simulink model "x64_32s_001_4ks"  (Simulink 7.4)
settings: Solver=ode45  StartTime=0.0  StopTime=100000  RelTol=1e-3
undefined symbols (not defined in the file): gcbh, ROACH, x64_32s_001_4ks_beam_mux_order_in_user_data_out, x64_32s_001_4ks_beam_mux_order_out_user_data_out, x64_32s_001_4ks_beam_mux_order_we_user_data_out, x64_32s_001_4ks_first_chan_user_data_out, x64_32s_001_4ks_gbe_dest_ip_user_data_out, x64_32s_001_4ks_gbe_dest_port_user_data_out, x64_32s_001_4ks_gbe_packet_cnt1_user_data_in, x64_32s_001_4ks_gbe_packet_cnt_user_data_in, x64_32s_001_4ks_heap_size_user_data_out, x64_32s_001_4ks_inst_seng_id0_user_data_in, x64_32s_001_4ks_last_chan_user_data_out, x64_32s_001_4ks_n_beams_user_data_out, x64_32s_001_4ks_qdr_in_cnt_user_data_in, x64_32s_001_4ks_status_user_data_in, x64_32s_001_4ks_x_window_mult_0_coeff_user_data_out, x64_32s_001_4ks_x_window_mult_1_coeff_user_data_out, x64_32s_001_4ks_x_window_mult_2_coeff_user_data_out, x64_32s_001_4ks_x_window_mult_3_coeff_user_data_out, x64_32s_001_4ks_xaui_cnt0_user_data_in, x64_32s_001_4ks_xaui_error_cnt0_user_data_in, x64_32s_001_4ks_xauibuf_out_cnt_user_data_in, x64_32s_001_4ks_xauibuf_window_cnt_user_data_in, x64_32s_001_4ks_y_window_mult_0_coeff_user_data_out, x64_32s_001_4ks_y_window_mult_1_coeff_user_data_out, x64_32s_001_4ks_y_window_mult_2_coeff_user_data_out, x64_32s_001_4ks_y_window_mult_3_coeff_user_data_out, x64_32s_001_4ks_y_window_mult_4_coeff_user_data_out, x64_32s_001_4ks_y_window_mult_5_coeff_user_data_out, x64_32s_001_4ks_y_window_mult_6_coeff_user_data_out, x64_32s_001_4ks_y_window_mult_7_coeff_user_data_out, xlBlockLoadCallback
libraries: xbsIndex_r4 x8559, casper_library_misc x308, casper_library_delays x33, casper_library_reorder x16, xps_library x5, ox_lib x1
(part 8 of 100)

== system x64_32s_001_4ks/mini_vacc ==
  new_acc : Inport
  In2 : Inport  Port=2
  In4 : Inport  Port=3
  In6 : Inport  Port=4
  In8 : Inport  Port=5
  In10 : Inport  Port=6
  In12 : Inport  Port=7
  In14 : Inport  Port=8
  In16 : Inport  Port=9
  In18 : Inport  Port=10
  In20 : Inport  Port=11
  In22 : Inport  Port=12
  In24 : Inport  Port=13
  In26 : Inport  Port=14
  In28 : Inport  Port=15
  In30 : Inport  Port=16
  In32 : Inport  Port=17
  simple_bram_vacc1 : Subsystem  AncestorBlock=ox_lib/simple_bram_vacc  mask(simple_bram_vacc){vec_len=8, arith_type=Unsigned, n_bits=32, bin_pt=0, acc_len=128}
      mask prompts: vec_len "Vector length:"; arith_type "Output type:"; n_bits "Bit width (output):"; bin_pt "Binary point (output):"; acc_len "Accumulation length?"
      mask help:
      | This simple vector accumulator is based on a shift register. It outputs its accumulated vector as soon as it is calculated.
      |
      | (I.e, it does not require a further new acc pulse, and therefore won't break if there are periods of invalid data)
      mask init:
      | simple_bram_vacc_init(gcb, ...
      |     'vec_len', vec_len, ...
      |     'arith_type', arith_type, ...
      |     'n_bits', n_bits, ...
      |     'bin_pt', bin_pt);
      | 
      | fmtstr = sprintf('Acc len: %d', acc_len);
      | set_param(gcb, 'AttributesFormatString', fmtstr);
      settings: AttributesFormatString=Acc len: 128
      mask StyleString: edit,popup(Signed|Unsigned),edit,edit,edit
  simple_bram_vacc10 : Subsystem  AncestorBlock=ox_lib/simple_bram_vacc  mask(simple_bram_vacc){vec_len=8, arith_type=Unsigned, n_bits=32, bin_pt=0, acc_len=128}
      mask prompts: vec_len "Vector length:"; arith_type "Output type:"; n_bits "Bit width (output):"; bin_pt "Binary point (output):"; acc_len "Accumulation length?"
      mask help:
      | This simple vector accumulator is based on a shift register. It outputs its accumulated vector as soon as it is calculated.
      |
      | (I.e, it does not require a further new acc pulse, and therefore won't break if there are periods of invalid data)
      mask init: same as x64_32s_001_4ks/mini_vacc/simple_bram_vacc1
      settings: AttributesFormatString=Acc len: 128
      mask StyleString: edit,popup(Signed|Unsigned),edit,edit,edit
  simple_bram_vacc11 : Subsystem  AncestorBlock=ox_lib/simple_bram_vacc  mask(simple_bram_vacc){vec_len=8, arith_type=Unsigned, n_bits=32, bin_pt=0, acc_len=128}
      mask prompts: vec_len "Vector length:"; arith_type "Output type:"; n_bits "Bit width (output):"; bin_pt "Binary point (output):"; acc_len "Accumulation length?"
      mask help:
      | This simple vector accumulator is based on a shift register. It outputs its accumulated vector as soon as it is calculated.
      |
      | (I.e, it does not require a further new acc pulse, and therefore won't break if there are periods of invalid data)
      mask init: same as x64_32s_001_4ks/mini_vacc/simple_bram_vacc1
      settings: AttributesFormatString=Acc len: 128
      mask StyleString: edit,popup(Signed|Unsigned),edit,edit,edit
  simple_bram_vacc12 : Subsystem  AncestorBlock=ox_lib/simple_bram_vacc  mask(simple_bram_vacc){vec_len=8, arith_type=Unsigned, n_bits=32, bin_pt=0, acc_len=128}
      mask prompts: vec_len "Vector length:"; arith_type "Output type:"; n_bits "Bit width (output):"; bin_pt "Binary point (output):"; acc_len "Accumulation length?"
      mask help:
      | This simple vector accumulator is based on a shift register. It outputs its accumulated vector as soon as it is calculated.
      |
      | (I.e, it does not require a further new acc pulse, and therefore won't break if there are periods of invalid data)
      mask init: same as x64_32s_001_4ks/mini_vacc/simple_bram_vacc1
      settings: AttributesFormatString=Acc len: 128
      mask StyleString: edit,popup(Signed|Unsigned),edit,edit,edit
  simple_bram_vacc13 : Subsystem  AncestorBlock=ox_lib/simple_bram_vacc  mask(simple_bram_vacc){vec_len=8, arith_type=Unsigned, n_bits=32, bin_pt=0, acc_len=128}
      mask prompts: vec_len "Vector length:"; arith_type "Output type:"; n_bits "Bit width (output):"; bin_pt "Binary point (output):"; acc_len "Accumulation length?"
      mask help:
      | This simple vector accumulator is based on a shift register. It outputs its accumulated vector as soon as it is calculated.
      |
      | (I.e, it does not require a further new acc pulse, and therefore won't break if there are periods of invalid data)
      mask init: same as x64_32s_001_4ks/mini_vacc/simple_bram_vacc1
      settings: AttributesFormatString=Acc len: 128
      mask StyleString: edit,popup(Signed|Unsigned),edit,edit,edit
  simple_bram_vacc14 : Subsystem  AncestorBlock=ox_lib/simple_bram_vacc  mask(simple_bram_vacc){vec_len=8, arith_type=Unsigned, n_bits=32, bin_pt=0, acc_len=128}
      mask prompts: vec_len "Vector length:"; arith_type "Output type:"; n_bits "Bit width (output):"; bin_pt "Binary point (output):"; acc_len "Accumulation length?"
      mask help:
      | This simple vector accumulator is based on a shift register. It outputs its accumulated vector as soon as it is calculated.
      |
      | (I.e, it does not require a further new acc pulse, and therefore won't break if there are periods of invalid data)
      mask init: same as x64_32s_001_4ks/mini_vacc/simple_bram_vacc1
      settings: AttributesFormatString=Acc len: 128
      mask StyleString: edit,popup(Signed|Unsigned),edit,edit,edit
  simple_bram_vacc15 : Subsystem  AncestorBlock=ox_lib/simple_bram_vacc  mask(simple_bram_vacc){vec_len=8, arith_type=Unsigned, n_bits=32, bin_pt=0, acc_len=128}
      mask prompts: vec_len "Vector length:"; arith_type "Output type:"; n_bits "Bit width (output):"; bin_pt "Binary point (output):"; acc_len "Accumulation length?"
      mask help:
      | This simple vector accumulator is based on a shift register. It outputs its accumulated vector as soon as it is calculated.
      |
      | (I.e, it does not require a further new acc pulse, and therefore won't break if there are periods of invalid data)
      mask init: same as x64_32s_001_4ks/mini_vacc/simple_bram_vacc1
      settings: AttributesFormatString=Acc len: 128
      mask StyleString: edit,popup(Signed|Unsigned),edit,edit,edit
  simple_bram_vacc16 : Subsystem  AncestorBlock=ox_lib/simple_bram_vacc  mask(simple_bram_vacc){vec_len=8, arith_type=Unsigned, n_bits=32, bin_pt=0, acc_len=128}
      mask prompts: vec_len "Vector length:"; arith_type "Output type:"; n_bits "Bit width (output):"; bin_pt "Binary point (output):"; acc_len "Accumulation length?"
      mask help:
      | This simple vector accumulator is based on a shift register. It outputs its accumulated vector as soon as it is calculated.
      |
      | (I.e, it does not require a further new acc pulse, and therefore won't break if there are periods of invalid data)
      mask init: same as x64_32s_001_4ks/mini_vacc/simple_bram_vacc1
      settings: AttributesFormatString=Acc len: 128
      mask StyleString: edit,popup(Signed|Unsigned),edit,edit,edit
  simple_bram_vacc2 : Subsystem  AncestorBlock=ox_lib/simple_bram_vacc  mask(simple_bram_vacc){vec_len=8, arith_type=Unsigned, n_bits=32, bin_pt=0, acc_len=128}
      mask prompts: vec_len "Vector length:"; arith_type "Output type:"; n_bits "Bit width (output):"; bin_pt "Binary point (output):"; acc_len "Accumulation length?"
      mask help:
      | This simple vector accumulator is based on a shift register. It outputs its accumulated vector as soon as it is calculated.
      |
      | (I.e, it does not require a further new acc pulse, and therefore won't break if there are periods of invalid data)
      mask init: same as x64_32s_001_4ks/mini_vacc/simple_bram_vacc1
      settings: AttributesFormatString=Acc len: 128
      mask StyleString: edit,popup(Signed|Unsigned),edit,edit,edit
  simple_bram_vacc3 : Subsystem  AncestorBlock=ox_lib/simple_bram_vacc  mask(simple_bram_vacc){vec_len=8, arith_type=Unsigned, n_bits=32, bin_pt=0, acc_len=128}
      mask prompts: vec_len "Vector length:"; arith_type "Output type:"; n_bits "Bit width (output):"; bin_pt "Binary point (output):"; acc_len "Accumulation length?"
      mask help:
      | This simple vector accumulator is based on a shift register. It outputs its accumulated vector as soon as it is calculated.
      |
      | (I.e, it does not require a further new acc pulse, and therefore won't break if there are periods of invalid data)
      mask init: same as x64_32s_001_4ks/mini_vacc/simple_bram_vacc1
      settings: AttributesFormatString=Acc len: 128
      mask StyleString: edit,popup(Signed|Unsigned),edit,edit,edit
  simple_bram_vacc4 : Subsystem  AncestorBlock=ox_lib/simple_bram_vacc  mask(simple_bram_vacc){vec_len=8, arith_type=Unsigned, n_bits=32, bin_pt=0, acc_len=128}
      mask prompts: vec_len "Vector length:"; arith_type "Output type:"; n_bits "Bit width (output):"; bin_pt "Binary point (output):"; acc_len "Accumulation length?"
      mask help:
      | This simple vector accumulator is based on a shift register. It outputs its accumulated vector as soon as it is calculated.
      |
      | (I.e, it does not require a further new acc pulse, and therefore won't break if there are periods of invalid data)
      mask init: same as x64_32s_001_4ks/mini_vacc/simple_bram_vacc1
      settings: AttributesFormatString=Acc len: 128
      mask StyleString: edit,popup(Signed|Unsigned),edit,edit,edit
  simple_bram_vacc5 : Subsystem  AncestorBlock=ox_lib/simple_bram_vacc  mask(simple_bram_vacc){vec_len=8, arith_type=Unsigned, n_bits=32, bin_pt=0, acc_len=128}
      mask prompts: vec_len "Vector length:"; arith_type "Output type:"; n_bits "Bit width (output):"; bin_pt "Binary point (output):"; acc_len "Accumulation length?"
      mask help:
      | This simple vector accumulator is based on a shift register. It outputs its accumulated vector as soon as it is calculated.
      |
      | (I.e, it does not require a further new acc pulse, and therefore won't break if there are periods of invalid data)
      mask init: same as x64_32s_001_4ks/mini_vacc/simple_bram_vacc1
      settings: AttributesFormatString=Acc len: 128
      mask StyleString: edit,popup(Signed|Unsigned),edit,edit,edit
  simple_bram_vacc6 : Subsystem  AncestorBlock=ox_lib/simple_bram_vacc  mask(simple_bram_vacc){vec_len=8, arith_type=Unsigned, n_bits=32, bin_pt=0, acc_len=128}
      mask prompts: vec_len "Vector length:"; arith_type "Output type:"; n_bits "Bit width (output):"; bin_pt "Binary point (output):"; acc_len "Accumulation length?"
      mask help:
      | This simple vector accumulator is based on a shift register. It outputs its accumulated vector as soon as it is calculated.
      |
      | (I.e, it does not require a further new acc pulse, and therefore won't break if there are periods of invalid data)
      mask init: same as x64_32s_001_4ks/mini_vacc/simple_bram_vacc1
      settings: AttributesFormatString=Acc len: 128
      mask StyleString: edit,popup(Signed|Unsigned),edit,edit,edit
  simple_bram_vacc7 : Subsystem  AncestorBlock=ox_lib/simple_bram_vacc  mask(simple_bram_vacc){vec_len=8, arith_type=Unsigned, n_bits=32, bin_pt=0, acc_len=128}
      mask prompts: vec_len "Vector length:"; arith_type "Output type:"; n_bits "Bit width (output):"; bin_pt "Binary point (output):"; acc_len "Accumulation length?"
      mask help:
      | This simple vector accumulator is based on a shift register. It outputs its accumulated vector as soon as it is calculated.
      |
      | (I.e, it does not require a further new acc pulse, and therefore won't break if there are periods of invalid data)
      mask init: same as x64_32s_001_4ks/mini_vacc/simple_bram_vacc1
      settings: AttributesFormatString=Acc len: 128
      mask StyleString: edit,popup(Signed|Unsigned),edit,edit,edit
  simple_bram_vacc8 : Subsystem  AncestorBlock=ox_lib/simple_bram_vacc  mask(simple_bram_vacc){vec_len=8, arith_type=Unsigned, n_bits=32, bin_pt=0, acc_len=128}
      mask prompts: vec_len "Vector length:"; arith_type "Output type:"; n_bits "Bit width (output):"; bin_pt "Binary point (output):"; acc_len "Accumulation length?"
      mask help:
      | This simple vector accumulator is based on a shift register. It outputs its accumulated vector as soon as it is calculated.
      |
      | (I.e, it does not require a further new acc pulse, and therefore won't break if there are periods of invalid data)
      mask init: same as x64_32s_001_4ks/mini_vacc/simple_bram_vacc1
      settings: AttributesFormatString=Acc len: 128
      mask StyleString: edit,popup(Signed|Unsigned),edit,edit,edit
  simple_bram_vacc9 : Subsystem  AncestorBlock=ox_lib/simple_bram_vacc  mask(simple_bram_vacc){vec_len=8, arith_type=Unsigned, n_bits=32, bin_pt=0, acc_len=128}
      mask prompts: vec_len "Vector length:"; arith_type "Output type:"; n_bits "Bit width (output):"; bin_pt "Binary point (output):"; acc_len "Accumulation length?"
      mask help:
      | This simple vector accumulator is based on a shift register. It outputs its accumulated vector as soon as it is calculated.
      |
      | (I.e, it does not require a further new acc pulse, and therefore won't break if there are periods of invalid data)
      mask init: same as x64_32s_001_4ks/mini_vacc/simple_bram_vacc1
      settings: AttributesFormatString=Acc len: 128
      mask StyleString: edit,popup(Signed|Unsigned),edit,edit,edit
  sync_out : Outport
  vld : Outport  Port=2
  Out1 : Outport  Port=3
  Out3 : Outport  Port=4
  Out5 : Outport  Port=5
  Out7 : Outport  Port=6
  Out9 : Outport  Port=7
  Out11 : Outport  Port=8
  Out13 : Outport  Port=9
  Out15 : Outport  Port=10
  Out17 : Outport  Port=11
  Out19 : Outport  Port=12
  Out21 : Outport  Port=13
  Out23 : Outport  Port=14
  Out25 : Outport  Port=15
  Out27 : Outport  Port=16
  Out29 : Outport  Port=17
  Out31 : Outport  Port=18
  wires:
  simple_bram_vacc16/1(dout) -> Out1/1
  In2/1 -> simple_bram_vacc16/2(din)
  simple_bram_vacc1/1(dout) -> Out3/1
  In4/1 -> simple_bram_vacc1/2(din)
  simple_bram_vacc1/2(valid) -> ?
  simple_bram_vacc2/1(dout) -> Out5/1
  In6/1 -> simple_bram_vacc2/2(din)
  simple_bram_vacc2/2(valid) -> ?
  simple_bram_vacc3/1(dout) -> Out7/1
  In8/1 -> simple_bram_vacc3/2(din)
  simple_bram_vacc3/2(valid) -> ?
  simple_bram_vacc4/1(dout) -> Out9/1
  In10/1 -> simple_bram_vacc4/2(din)
  simple_bram_vacc4/2(valid) -> ?
  simple_bram_vacc5/1(dout) -> Out11/1
  In12/1 -> simple_bram_vacc5/2(din)
  simple_bram_vacc5/2(valid) -> ?
  simple_bram_vacc6/1(dout) -> Out13/1
  In14/1 -> simple_bram_vacc6/2(din)
  simple_bram_vacc6/2(valid) -> ?
  simple_bram_vacc7/1(dout) -> Out15/1
  In16/1 -> simple_bram_vacc7/2(din)
  simple_bram_vacc7/2(valid) -> ?
  simple_bram_vacc8/1(dout) -> Out17/1
  In18/1 -> simple_bram_vacc8/2(din)
  simple_bram_vacc8/2(valid) -> ?
  simple_bram_vacc9/1(dout) -> Out19/1
  In20/1 -> simple_bram_vacc9/2(din)
  simple_bram_vacc9/2(valid) -> ?
  simple_bram_vacc10/1(dout) -> Out21/1
  In22/1 -> simple_bram_vacc10/2(din)
  simple_bram_vacc10/2(valid) -> ?
  simple_bram_vacc11/1(dout) -> Out23/1
  In24/1 -> simple_bram_vacc11/2(din)
  simple_bram_vacc11/2(valid) -> ?
  simple_bram_vacc12/1(dout) -> Out25/1
  In26/1 -> simple_bram_vacc12/2(din)
  simple_bram_vacc12/2(valid) -> ?
  simple_bram_vacc13/1(dout) -> Out27/1
  In28/1 -> simple_bram_vacc13/2(din)
  simple_bram_vacc13/2(valid) -> ?
  simple_bram_vacc14/1(dout) -> Out29/1
  In30/1 -> simple_bram_vacc14/2(din)
  simple_bram_vacc14/2(valid) -> ?
  simple_bram_vacc15/1(dout) -> Out31/1
  In32/1 -> simple_bram_vacc15/2(din)
  simple_bram_vacc15/2(valid) -> ?
  simple_bram_vacc16/2(valid) -> vld/1
  new_acc/1 -> simple_bram_vacc14/1(new_acc), simple_bram_vacc15/1(new_acc), simple_bram_vacc13/1(new_acc), simple_bram_vacc12/1(new_acc), simple_bram_vacc11/1(new_acc), simple_bram_vacc10/1(new_acc), simple_bram_vacc9/1(new_acc), simple_bram_vacc8/1(new_acc), simple_bram_vacc7/1(new_acc), simple_bram_vacc6/1(new_acc), simple_bram_vacc5/1(new_acc), simple_bram_vacc4/1(new_acc), simple_bram_vacc3/1(new_acc), simple_bram_vacc2/1(new_acc), simple_bram_vacc1/1(new_acc), simple_bram_vacc16/1(new_acc)
  simple_bram_vacc16/3(sync) -> sync_out/1

== system x64_32s_001_4ks/mini_vacc_mux1 ==
  sync : Inport
  din : Inport  Port=2
  vld_in : Inport  Port=3
  Counter : xbsIndex_r4/Counter  cnt_type=Free Running  cnt_to=Inf  operation=Up  start_count=0  cnt_by_val=1  arith_type=Unsigned  n_bits=vec_len+4  bin_pt=0  load_pin=off  rst=on  en=off  explicit_period=on  period=1  dbl_ovrd=off  use_behavioral_HDL=off  implementation=Fabric  block_type=counter
      settings: block_version=11.4
  Delay : xbsIndex_r4/Delay  en=off  latency=2  dbl_ovrd=off  reg_retiming=off  block_type=delay
      settings: block_version=11.4
  Delay1 : xbsIndex_r4/Delay  en=off  latency=1  dbl_ovrd=off  reg_retiming=off  block_type=delay
      settings: block_version=11.4
  Delay2 : xbsIndex_r4/Delay  en=off  latency=1  dbl_ovrd=off  reg_retiming=off  block_type=delay
      settings: block_version=11.4
  Delay4 : xbsIndex_r4/Delay  en=off  latency=1  dbl_ovrd=off  reg_retiming=off  block_type=delay
      settings: block_version=11.4
  Mux : xbsIndex_r4/Mux  inputs=16  en=off  latency=2  precision=Full  arith_type=Unsigned  n_bits=16  bin_pt=14  quantization=Truncate  overflow=Wrap  dbl_ovrd=off  block_type=mux
      settings: block_version=11.4
  Single Port RAM{1..15} (15 alike) : xbsIndex_r4/Single Port RAM  depth=8  initVector=sin(pi*(0:15)/16)  distributed_mem=Distributed memory  write_mode=Read Before Write  rst=off  init_reg=0  en=off  latency=1  dbl_ovrd=off  optimize=Area  use_rpm=on  block_type=spram
  Slice : xbsIndex_r4/Slice  nbits=4  boolean_output=off  mode=Lower Bit Location + Width  bit1=0  base1=MSB of Input  bit0=vec_len  base0=LSB of Input  dbl_ovrd=off  block_type=slice
  Slice1 : xbsIndex_r4/Slice  nbits=vec_len  boolean_output=off  mode=Lower Bit Location + Width  bit1=0  base1=MSB of Input  bit0=0  base0=LSB of Input  dbl_ovrd=off  block_type=slice
  pulse_ext : casper_library_misc/pulse_ext  SystemSampleTime=-1  pulse_len=8*16
  uncram2 : Subsystem  AncestorBlock=gavrt_library/uncram  mask(uncram){num_slice=16, slice_width=32, bin_pt=0, arith_type=0}
      mask prompts: num_slice "Number of slices"; slice_width "Slice Width"; bin_pt "Output Binary Point"; arith_type "Output Arithmetic Type (0=Unsigned, 1=Signed)"
      mask help: Takes a concatenated input and slices it up into even pieces and reinterprets them as signed fixed point numbers with a given binary point.
      mask init:
      | uncram_init(gcb, ...
      |     'num_slice', num_slice, ...
      |     'slice_width', slice_width, ...
      |     'bin_pt', bin_pt, ...
      |     'arith_type', arith_type);
      settings: AttributesFormatString=UFix_32_0
  dout : Outport
  sync_out : Outport  Port=2
  vld : Outport  Port=3
  wires:
  Delay/1 -> sync_out/1, pulse_ext/1
  Slice/1 -> Delay2/1
  Counter/1 -> Slice/1, Slice1/1
  Mux/1 -> dout/1
  din/1 -> uncram2/1(In)
  sync/1 -> Counter/1, Delay1/1
  Delay2/1 -> Mux/1
  Delay1/1 -> Delay/1
  Single Port RAM1/1 -> Mux/3
  Single Port RAM2/1 -> Mux/4
  Single Port RAM3/1 -> Mux/5
  Single Port RAM4/1 -> Mux/6
  Single Port RAM5/1 -> Mux/7
  Single Port RAM6/1 -> Mux/8
  Single Port RAM7/1 -> Mux/9
  Single Port RAM8/1 -> Mux/10
  Single Port RAM9/1 -> Mux/11
  Single Port RAM10/1 -> Mux/12
  Single Port RAM11/1 -> Mux/13
  Single Port RAM12/1 -> Mux/14
  Single Port RAM13/1 -> Mux/15
  Single Port RAM14/1 -> Mux/16
  Single Port RAM15/1 -> Mux/17
  Slice1/1 -> Single Port RAM2/1, Single Port RAM3/1, Single Port RAM4/1, Single Port RAM5/1, Single Port RAM6/1, Single Port RAM7/1, Single Port RAM8/1, Single Port RAM9/1, Single Port RAM10/1, Single Port RAM11/1, Single Port RAM12/1, Single Port RAM13/1, Single Port RAM14/1, Single Port RAM15/1, Single Port RAM1/1
  vld_in/1 -> Single Port RAM1/3, Single Port RAM2/3, Single Port RAM3/3, Single Port RAM4/3, Single Port RAM5/3, Single Port RAM6/3, Single Port RAM7/3, Single Port RAM8/3, Single Port RAM9/3, Single Port RAM10/3, Single Port RAM11/3, Single Port RAM12/3, Single Port RAM13/3, Single Port RAM14/3, Single Port RAM15/3
  uncram2/1(Out1) -> Delay4/1
  uncram2/2(Out2) -> Single Port RAM1/2
  uncram2/3(Out3) -> Single Port RAM2/2
  uncram2/4(Out4) -> Single Port RAM3/2
  uncram2/5(Out5) -> Single Port RAM4/2
  uncram2/6(Out6) -> Single Port RAM5/2
  uncram2/7(Out7) -> Single Port RAM6/2
  uncram2/8(Out8) -> Single Port RAM7/2
  uncram2/9(Out9) -> Single Port RAM8/2
  uncram2/10(Out10) -> Single Port RAM9/2
  uncram2/11(Out11) -> Single Port RAM10/2
  uncram2/12(Out12) -> Single Port RAM11/2
  uncram2/13(Out13) -> Single Port RAM12/2
  uncram2/14(Out14) -> Single Port RAM13/2
  uncram2/15(Out15) -> Single Port RAM14/2
  uncram2/16(Out16) -> Single Port RAM15/2
  Delay4/1 -> Mux/2
  pulse_ext/1 -> vld/1

== system x64_32s_001_4ks/n_beams ==
  sim_in : Inport
  x64_32s_001_4ks_n_beams_user_data_out : xbsIndex_r4/Gateway In  arith_type=Unsigned  n_bits=32  bin_pt=0  quantization="Round  (unbiased: +/- Inf)"  overflow=Saturate  period=1  dbl_ovrd=off  timing_constraint=None  locs_specified=off  LOCs={}  hdl_port=on  block_type=gatewayin
      settings: infoedit="Gateway in block.  Converts inputs of type Simulink integer, double and fixed point to  Xilinx fixed point type.<br><br>Hardware notes:  In hardware these blocks become top level input ports."  block_version=11.4
  reg_in : Outport
  wires:
  sim_in/1 -> x64_32s_001_4ks_n_beams_user_data_out/1
  x64_32s_001_4ks_n_beams_user_data_out/1 -> reg_in/1

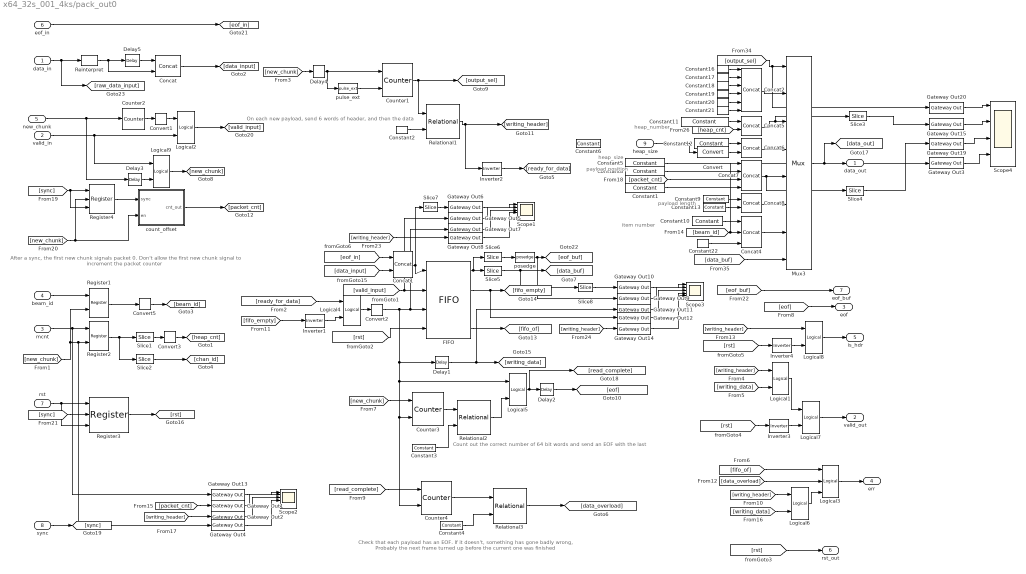
== system x64_32s_001_4ks/pack_out0 ==
  note: heap_size
  note: Count out the correct
      | number of 64 bit words
      | and send an EOF
      | with the last
  note: Check that each payload has an EOF. If it doesn't, something has gone badly wrong,
      | Probably the next frame turned up before the current one was finished
  note: On each new payload,
      | send 6 words of header, and
      | then the data
  note: heap_number
  note: payload
      | length
  note: item
      | number
  note: payload
      | position
  note: After a sync, the first new chunk
      | signals packet 0.
      | Don't allow the first new chunk signal
      | to increment the packet counter
  data_in : Inport  OutDataType=sfix(16)  OutScaling=2^0
  valid_in : Inport  Port=2  OutDataType=sfix(16)  OutScaling=2^0
  mcnt : Inport  Port=3  OutDataType=sfix(16)  OutScaling=2^0
  beam_id : Inport  Port=4  OutDataType=sfix(16)  OutScaling=2^0
  new_chunk : Inport  Port=5  OutDataType=sfix(16)  OutScaling=2^0
  eof_in : Inport  Port=6  OutDataType=sfix(16)  OutScaling=2^0
  rst : Inport  Port=7  OutDataType=sfix(16)  OutScaling=2^0
  sync : Inport  Port=8  OutDataType=sfix(16)  OutScaling=2^0
  heap_size : Inport  Port=9  OutDataType=sfix(16)  OutScaling=2^0
  Concat{,1,5..6,8} (5 alike) : xbsIndex_r4/Concat  num_inputs=2  dbl_ovrd=off  block_type=concat
      settings: block_version=10.1.3
  Concat2 : xbsIndex_r4/Concat  num_inputs=6  dbl_ovrd=off  block_type=concat
      settings: block_version=10.1.3
  Concat4 : xbsIndex_r4/Concat  num_inputs=3  dbl_ovrd=off  block_type=concat
      settings: block_version=10.1.3
  Concat7 : xbsIndex_r4/Concat  num_inputs=4  dbl_ovrd=off  block_type=concat
      settings: block_version=10.1.3
  Constant1 : xbsIndex_r4/Constant  arith_type=Unsigned  const=0  n_bits=n_payload  bin_pt=0  explicit_period=on  period=1  equ=P=C  opselect=C  inp2=PCIN>>17  opr=+  inp1=P  carry=CIN  dbl_ovrd=off  block_type=constant
  Constant10 : xbsIndex_r4/Constant  arith_type=Unsigned  const=spead_item  n_bits=spead_msw-spead_lsw-n_beams  bin_pt=0  explicit_period=on  period=1  equ=P=C  opselect=C  inp2=PCIN>>17  opr=+  inp1=P  carry=CIN  dbl_ovrd=off  block_type=constant
  Constant11 : xbsIndex_r4/Constant  arith_type=Unsigned  const=1 + 2^(spead_msw-spead_lsw-1)  n_bits=spead_msw-spead_lsw  bin_pt=0  explicit_period=on  period=1  equ=P=C  opselect=C  inp2=PCIN>>17  opr=+  inp1=P  carry=CIN  dbl_ovrd=off  block_type=constant
  Constant12 : xbsIndex_r4/Constant  arith_type=Unsigned  const="2 +  + 2^(spead_msw-spead_lsw-1)"  n_bits=spead_msw-spead_lsw  bin_pt=0  explicit_period=on  period=1  equ=P=C  opselect=C  inp2=PCIN>>17  opr=+  inp1=P  carry=CIN  dbl_ovrd=off  block_type=constant
  Constant13 : xbsIndex_r4/Constant  arith_type=Unsigned  const=2^n_payload  n_bits=spead_lsw  bin_pt=0  explicit_period=on  period=1  equ=P=C  opselect=C  inp2=PCIN>>17  opr=+  inp1=P  carry=CIN  dbl_ovrd=off  block_type=constant
  Constant16 : xbsIndex_r4/Constant  arith_type=Unsigned  const=0x53  n_bits=8  bin_pt=0  explicit_period=on  period=1  equ=P=C  opselect=C  inp2=PCIN>>17  opr=+  inp1=P  carry=CIN  dbl_ovrd=off  block_type=constant
  Constant17 : xbsIndex_r4/Constant  arith_type=Unsigned  const=4  n_bits=8  bin_pt=0  explicit_period=on  period=1  equ=P=C  opselect=C  inp2=PCIN>>17  opr=+  inp1=P  carry=CIN  dbl_ovrd=off  block_type=constant
  Constant18 : xbsIndex_r4/Constant  arith_type=Unsigned  const=(spead_msw-spead_lsw)/8  n_bits=8  bin_pt=0  explicit_period=on  period=1  equ=P=C  opselect=C  inp2=PCIN>>17  opr=+  inp1=P  carry=CIN  dbl_ovrd=off  block_type=constant
  Constant19 : xbsIndex_r4/Constant  arith_type=Unsigned  const=spead_lsw/8  n_bits=8  bin_pt=0  explicit_period=on  period=1  equ=P=C  opselect=C  inp2=PCIN>>17  opr=+  inp1=P  carry=CIN  dbl_ovrd=off  block_type=constant
  Constant2 : xbsIndex_r4/Constant  arith_type=Unsigned  const=6  n_bits=ceil(log2(6))  bin_pt=0  explicit_period=on  period=1  equ=P=C  opselect=C  inp2=PCIN>>17  opr=+  inp1=P  carry=CIN  dbl_ovrd=off  block_type=constant
  Constant20 : xbsIndex_r4/Constant  arith_type=Unsigned  const=0  n_bits=16  bin_pt=0  explicit_period=on  period=1  equ=P=C  opselect=C  inp2=PCIN>>17  opr=+  inp1=P  carry=CIN  dbl_ovrd=off  block_type=constant
  Constant21 : xbsIndex_r4/Constant  arith_type=Unsigned  const=5  n_bits=16  bin_pt=0  explicit_period=on  period=1  equ=P=C  opselect=C  inp2=PCIN>>17  opr=+  inp1=P  carry=CIN  dbl_ovrd=off  block_type=constant
  Constant22 : xbsIndex_r4/Constant  arith_type=Unsigned  const=0  n_bits=spead_lsw  bin_pt=0  explicit_period=on  period=1  equ=P=C  opselect=C  inp2=PCIN>>17  opr=+  inp1=P  carry=CIN  dbl_ovrd=off  block_type=constant
  Constant3 : xbsIndex_r4/Constant  arith_type=Unsigned  const=2^(n_payload-3)-1  n_bits=n_payload-3  bin_pt=0  explicit_period=on  period=1  equ=P=C  opselect=C  inp2=PCIN>>17  opr=+  inp1=P  carry=CIN  dbl_ovrd=off  block_type=constant
  Constant4 : xbsIndex_r4/Constant  arith_type=Unsigned  const=2^(n_payload-3)-1  n_bits=n_payload-3  bin_pt=0  explicit_period=on  period=1  equ=P=C  opselect=C  inp2=PCIN>>17  opr=+  inp1=P  carry=CIN  dbl_ovrd=off  block_type=constant
  Constant5 : xbsIndex_r4/Constant  arith_type=Unsigned  const=3 + 2^(spead_msw-spead_lsw-1)  n_bits=spead_msw-spead_lsw  bin_pt=0  explicit_period=on  period=1  equ=P=C  opselect=C  inp2=PCIN>>17  opr=+  inp1=P  carry=CIN  dbl_ovrd=off  block_type=constant
  Constant6 : xbsIndex_r4/Constant  arith_type=Unsigned  const=2^(n_samples+n_chans + 2)  n_bits=spead_lsw  bin_pt=0  explicit_period=on  period=1  equ=P=C  opselect=C  inp2=PCIN>>17  opr=+  inp1=P  carry=CIN  dbl_ovrd=off  block_type=constant
  Constant8 : xbsIndex_r4/Constant  arith_type=Unsigned  const=0  n_bits=spead_lsw- (n_chans+n_samples+2 - n_payload) - n_payload  bin_pt=0  explicit_period=on  period=1  equ=P=C  opselect=C  inp2=PCIN>>17  opr=+  inp1=P  carry=CIN  dbl_ovrd=off  block_type=constant
  Constant9 : xbsIndex_r4/Constant  arith_type=Unsigned  const=4 + 2^(spead_msw-spead_lsw-1)  n_bits=spead_msw-spead_lsw  bin_pt=0  explicit_period=on  period=1  equ=P=C  opselect=C  inp2=PCIN>>17  opr=+  inp1=P  carry=CIN  dbl_ovrd=off  block_type=constant
  Convert : xbsIndex_r4/Convert  arith_type=Unsigned  n_bits=spead_lsw  bin_pt=0  quantization=Truncate  overflow=Wrap  latency=1  dbl_ovrd=off  pipeline=off  block_type=convert
      settings: block_version=11.4
  Convert1 : xbsIndex_r4/Convert  arith_type=Boolean  n_bits=1  bin_pt=0  quantization=Truncate  overflow=Wrap  latency=0  dbl_ovrd=off  pipeline=off  block_type=convert
      settings: block_version=11.4
  Convert2 : xbsIndex_r4/Convert  arith_type=Boolean  n_bits=1  bin_pt=0  quantization=Truncate  overflow=Wrap  latency=0  dbl_ovrd=off  pipeline=off  block_type=convert
      settings: block_version=11.4
  Convert3 : xbsIndex_r4/Convert  arith_type=Unsigned  n_bits=spead_lsw  bin_pt=0  quantization=Truncate  overflow=Wrap  latency=0  dbl_ovrd=off  pipeline=off  block_type=convert
      settings: block_version=11.4
  Convert5 : xbsIndex_r4/Convert  arith_type=Unsigned  n_bits=n_beams  bin_pt=0  quantization=Truncate  overflow=Wrap  latency=0  dbl_ovrd=off  pipeline=off  block_type=convert
      settings: block_version=11.4
  Counter1 : xbsIndex_r4/Counter  cnt_type=Count Limited  cnt_to=6  operation=Up  start_count=0  cnt_by_val=1  arith_type=Unsigned  n_bits=ceil(log2(6))  bin_pt=0  load_pin=off  rst=on  en=on  explicit_period=on  period=1  dbl_ovrd=off  use_behavioral_HDL=off  implementation=Fabric  use_rpm=on  block_type=counter
      settings: xl_area=[0, 0, 0, 0, 0, 0, 0]
  Counter2 : xbsIndex_r4/Counter  cnt_type=Free Running  cnt_to=1023  operation=Up  start_count=0  cnt_by_val=1  arith_type=Unsigned  n_bits=1  bin_pt=0  load_pin=off  rst=on  en=off  explicit_period=on  period=1  dbl_ovrd=off  use_behavioral_HDL=off  implementation=Fabric  use_rpm=on  block_type=counter
      settings: xl_area=[0, 0, 0, 0, 0, 0, 0]
  Counter3 : xbsIndex_r4/Counter  cnt_type=Count Limited  cnt_to=2^(n_payload-3)-1  operation=Up  start_count=0  cnt_by_val=1  arith_type=Unsigned  n_bits=n_payload-3  bin_pt=0  load_pin=off  rst=on  en=on  explicit_period=on  period=1  dbl_ovrd=off  use_behavioral_HDL=off  implementation=Fabric  use_rpm=on  block_type=counter
      settings: xl_area=[0, 0, 0, 0, 0, 0, 0]
  Counter4 : xbsIndex_r4/Counter  cnt_type=Free Running  cnt_to=1  operation=Up  start_count=0  cnt_by_val=1  arith_type=Unsigned  n_bits=10  bin_pt=0  load_pin=off  rst=on  en=on  explicit_period=on  period=1  dbl_ovrd=off  use_behavioral_HDL=off  implementation=Fabric  use_rpm=on  block_type=counter
      settings: xl_area=[0, 0, 0, 0, 0, 0, 0]
  Delay{1..5} (5 alike) : xbsIndex_r4/Delay  en=off  latency=1  dbl_ovrd=off  reg_retiming=on  block_type=delay
      settings: block_version=11.4
  FIFO : xbsIndex_r4/FIFO  depth=16  percent_nbits=1  rst=on  en=off  use_dcount=off  use_almost_empty=off  almost_empty_thresh=2  use_almost_full=off  almost_full_thresh=14  dbl_ovrd=off  mem_type=Distributed RAM  explicit_period=off  block_type=fifo
      settings: block_version=11.4
  Gateway Out{1..15,19..20} (17 alike) : xbsIndex_r4/Gateway Out  hdl_port=off  timing_constraint=None  locs_specified=off  LOCs={}  block_type=gatewayout
      settings: infoedit="Gateway out block.  Converts Xilinx fixed point inputs into ouputs of type Simulink integer, double, or fixed point.<br><br>Hardware notes:  In hardware these blocks become top level output ports or are discarded, depending on how they are configured."  block_version=11.4
  Inverter{1..4} (4 alike) : xbsIndex_r4/Inverter  en=off  latency=0  dbl_ovrd=off  block_type=inv
      settings: block_version=11.4
  Logical1 : xbsIndex_r4/Logical  logical_function=OR  inputs=2  en=off  latency=0  precision=Full  arith_type=Unsigned  n_bits=8  bin_pt=2  align_bp=on  dbl_ovrd=off  block_type=logical
      settings: xl_area=[0, 0, 0, 0, 0, 0, 0]
  Logical{2,4..9} (7 alike) : xbsIndex_r4/Logical  logical_function=AND  inputs=2  en=off  latency=0  precision=Full  arith_type=Unsigned  n_bits=8  bin_pt=2  align_bp=on  dbl_ovrd=off  block_type=logical
      settings: xl_area=[0, 0, 0, 0, 0, 0, 0]
  Logical3 : xbsIndex_r4/Logical  logical_function=OR  inputs=3  en=off  latency=0  precision=Full  arith_type=Unsigned  n_bits=8  bin_pt=2  align_bp=on  dbl_ovrd=off  block_type=logical
      settings: xl_area=[0, 0, 0, 0, 0, 0, 0]
  Mux3 : xbsIndex_r4/Mux  inputs=7  en=off  latency=0  precision=Full  arith_type="Signed  (2's comp)"  n_bits=8  bin_pt=2  quantization=Truncate  overflow=Wrap  dbl_ovrd=off  block_type=mux
      settings: xl_area=[0, 0, 0, 0, 0, 0, 0]
  Register1 : xbsIndex_r4/Register  init=0  rst=off  en=on  dbl_ovrd=off  block_type=register
  Register2 : xbsIndex_r4/Register  init=0  rst=off  en=on  dbl_ovrd=off  block_type=register
  Register3 : xbsIndex_r4/Register  init=0  rst=on  en=on  dbl_ovrd=off  block_type=register
  Register4 : xbsIndex_r4/Register  init=0  rst=on  en=on  dbl_ovrd=off  block_type=register
  Reinterpret : xbsIndex_r4/Reinterpret  force_arith_type=on  arith_type=Unsigned  force_bin_pt=on  bin_pt=0  block_type=reinterpret
      settings: infoedit="Changes signal type without altering the binary representation.   You can changed the signal between signed and unsigned, and relocate the binary point.<br><br>Hardware notes: In hardware this block costs nothing.<br><br>Example:  Suppose the input is 6 bits wide, signed, with 2 fractional bits, and the output is forced to unsigned with 0 fractional bits.  Then an input of -2.0 (1110.00 in binary 2's complement) becomes an output of 56 (111000 in binary)."
  Relational1 : xbsIndex_r4/Relational  mode=a<b  en=off  latency=0  dbl_ovrd=off  block_type=relational
      settings: xl_area=[0, 0, 0, 0, 0, 0, 0]  block_version=10.1.3
  Relational2 : xbsIndex_r4/Relational  mode=a=b  en=off  latency=0  dbl_ovrd=off  block_type=relational
      settings: xl_area=[0, 0, 0, 0, 0, 0, 0]  block_version=10.1.3
  Relational3 : xbsIndex_r4/Relational  mode=a>b  en=off  latency=0  dbl_ovrd=off  block_type=relational
      settings: xl_area=[0, 0, 0, 0, 0, 0, 0]  block_version=10.1.3
  Scope1 : Scope  NumInputPorts=6
      settings: YMin=-5~-5~-5~-5~-5~-5  YMax=5~5~5~5~5~5  SaveName=ScopeData8
  Scope2 : Scope  NumInputPorts=6
      settings: YMin=-5~-5~-5~-5~-5~-5  YMax=5~5~5~5~5~5  SaveName=ScopeData6
  Scope3 : Scope  NumInputPorts=6
      settings: YMin=-5~-5~-5~-5~-5~-5  YMax=5~5~5~5~5~5  SaveName=ScopeData9
  Scope4 : Scope  NumInputPorts=8
      settings: YMin=-5~-5~-5~-5~-5~-5~-5~-5  YMax=5~5~5~5~5~5~5~5  SaveName=ScopeData5
  Slice1 : xbsIndex_r4/Slice  nbits=48-n_chans  boolean_output=off  mode=Lower Bit Location + Width  bit1=0  base1=MSB of Input  bit0=n_chans  base0=LSB of Input  dbl_ovrd=off  block_type=slice
  Slice2 : xbsIndex_r4/Slice  nbits=n_chans  boolean_output=off  mode=Lower Bit Location + Width  bit1=0  base1=MSB of Input  bit0=0  base0=LSB of Input  dbl_ovrd=off  block_type=slice
  Slice3 : xbsIndex_r4/Slice  nbits=40  boolean_output=off  mode=Lower Bit Location + Width  bit1=0  base1=MSB of Input  bit0=0  base0=LSB of Input  dbl_ovrd=off  block_type=slice
  Slice4 : xbsIndex_r4/Slice  nbits=40  boolean_output=off  mode=Lower Bit Location + Width  bit1=0  base1=MSB of Input  bit0=0  base0=LSB of Input  dbl_ovrd=off  block_type=slice
  Slice5 : xbsIndex_r4/Slice  nbits=64  boolean_output=off  mode=Lower Bit Location + Width  bit1=0  base1=MSB of Input  bit0=0  base0=LSB of Input  dbl_ovrd=off  block_type=slice
  Slice6 : xbsIndex_r4/Slice  nbits=1  boolean_output=on  mode=Lower Bit Location + Width  bit1=0  base1=MSB of Input  bit0=64  base0=LSB of Input  dbl_ovrd=off  block_type=slice
  Slice7 : xbsIndex_r4/Slice  nbits=32  boolean_output=off  mode=Lower Bit Location + Width  bit1=0  base1=MSB of Input  bit0=0  base0=LSB of Input  dbl_ovrd=off  block_type=slice
  Slice8 : xbsIndex_r4/Slice  nbits=32  boolean_output=off  mode=Lower Bit Location + Width  bit1=0  base1=MSB of Input  bit0=0  base0=LSB of Input  dbl_ovrd=off  block_type=slice
  count_offset : Subsystem  mask{output_width=n_chans+n_samples+2 - n_payload, offset=0}
      mask prompts: output_width "output bit width"; offset "lsb offset (2^?)"
  posedge : casper_library_misc/posedge  Tag=Rising Edge Detector  SystemSampleTime=-1
  pulse_ext : casper_library_misc/pulse_ext  SystemSampleTime=-1  pulse_len=6
  data_out : Outport  OutDataType=sfix(16)  OutScaling=2^0
  valid_out : Outport  Port=2  OutDataType=sfix(16)  OutScaling=2^0
  eof : Outport  Port=3  OutDataType=sfix(16)  OutScaling=2^0
  err : Outport  Port=4  OutDataType=sfix(16)  OutScaling=2^0
  is_hdr : Outport  Port=5  OutDataType=sfix(16)  OutScaling=2^0
  rst_out : Outport  Port=6  OutDataType=sfix(16)  OutScaling=2^0
  eof_buf : Outport  Port=7  OutDataType=sfix(16)  OutScaling=2^0
  wires:
  Constant9/1 -> Concat8/1
  Constant13/1 -> Concat8/2
  Concat6/1 -> Mux3/4
  Constant12/1 -> Concat6/1
  Concat5/1 -> Mux3/3, Slice3/1
  [heap_cnt] -> Concat5/2
  Constant11/1 -> Concat5/1
  Constant10/1 -> Concat4/1
  Constant21/1 -> Concat2/6
  Constant20/1 -> Concat2/5
  Constant19/1 -> Concat2/4
  Constant18/1 -> Concat2/3
  Constant17/1 -> Concat2/2
  Constant16/1 -> Concat2/1
  Concat2/1 -> Mux3/2
  [output_sel] -> Mux3/1, Gateway Out20/1
  Mux3/1 -> data_out/1, [data_out]
  Delay5/1 -> Concat/1
  Reinterpret/1 -> Delay5/1, Concat/2
  data_in/1 -> [raw_data_input], Reinterpret/1
  Concat/1 -> [data_input]
  Concat8/1 -> Mux3/6
  Concat4/1 -> Mux3/7
  [data_buf] -> Mux3/8
  new_chunk/1 -> Counter2/1, Delay3/1
  Counter2/1 -> Convert1/1
  valid_in/1 -> Logical2/2, Logical9/1
  Logical2/1 -> [valid_input]
  [valid_input] -> FIFO/2, Gateway Out5/1
  Slice1/1 -> Convert3/1
  Slice2/1 -> [chan_id]
  mcnt/1 -> Register2/1, Gateway Out13/1
  Register2/1 -> Slice1/1, Slice2/1
  [new_chunk] -> Register1/2, Register2/2, Gateway Out4/1
  Concat7/1 -> Mux3/5, Slice4/1
  Counter1/1 -> [output_sel], Relational1/1
  [new_chunk] -> Delay4/1
  pulse_ext/1 -> Counter1/2
  Constant2/1 -> Relational1/2
  Relational1/1 -> [writing_header], Inverter2/1
  [writing_header] -> Logical1/1
  [writing_data] -> Logical1/2
  FIFO/4 -> [fifo_of]
  [fifo_of] -> Logical3/1
  Logical3/1 -> err/1
  FIFO/2 -> [fifo_empty], Gateway Out12/1
  [fifo_empty] -> Inverter1/1
  Logical4/1 -> Convert2/1
  [ready_for_data] -> Logical4/1
  Delay1/1 -> [writing_data], Gateway Out11/1
  [new_chunk] -> Counter3/1
  Counter3/1 -> Relational2/1
  Constant3/1 -> Relational2/2
  Relational2/1 -> Logical5/2
  Logical5/1 -> Delay2/1, [read_complete]
  Delay2/1 -> [eof]
  [eof] -> eof/1
  [data_overload] -> Logical3/2
  Convert1/1 -> Logical2/1
  Inverter1/1 -> Logical4/2
  Inverter2/1 -> [ready_for_data]
  Convert2/1 -> Delay1/1, Counter3/2, Counter4/2, Logical5/1, FIFO/3, Gateway Out7/1
  Convert3/1 -> [heap_cnt]
  [rst] -> FIFO/4
  rst/1 -> Register3/1, Register3/3
  Register3/1 -> [rst]
  [rst] -> rst_out/1
  Logical1/1 -> Logical7/1
  Inverter3/1 -> Logical7/2
  Logical7/1 -> valid_out/1
  [rst] -> Inverter3/1
  [read_complete] -> Counter4/1
  Counter4/1 -> Relational3/1
  Constant4/1 -> Relational3/2
  Relational3/1 -> [data_overload]
  Logical6/1 -> Logical3/3
  [writing_header] -> Logical6/1
  [writing_data] -> Logical6/2
  Delay4/1 -> Counter1/1, pulse_ext/1
  Inverter4/1 -> Logical8/2
  [rst] -> Inverter4/1
  [writing_header] -> Logical8/1
  Logical8/1 -> is_hdr/1
  Convert5/1 -> [beam_id]
  Constant22/1 -> Concat4/3
  [beam_id] -> Concat4/2, Gateway Out3/1
  beam_id/1 -> Register1/1
  Register1/1 -> Convert5/1
  Gateway Out13/1 -> Scope2/1
  Gateway Out1/1 -> Scope2/2
  Gateway Out2/1 -> Scope2/3
  Gateway Out4/1 -> Scope2/4
  [packet_cnt] -> Gateway Out1/1
  [writing_header] -> Gateway Out2/1
  Constant5/1 -> Concat7/1
  [sync] -> Register4/1, Register4/3
  count_offset/1(cnt_out) -> [packet_cnt]
  sync/1 -> [sync]
  [sync] -> Register3/2
  Gateway Out20/1 -> Scope4/1
  ? -> Scope4/2
  Gateway Out15/1 -> Scope4/3
  ? -> Scope4/4
  Gateway Out19/1 -> Scope4/5
  ? -> Scope4/6
  Gateway Out3/1 -> Scope4/7
  Slice3/1 -> Gateway Out15/1
  Constant8/1 -> Concat7/2
  [packet_cnt] -> Concat7/3
  Constant1/1 -> Concat7/4
  Slice4/1 -> Gateway Out19/1
  Concat1/1 -> FIFO/1, Slice7/1
  [eof_in] -> Concat1/1
  [data_input] -> Concat1/2
  FIFO/1 -> Slice5/1, Slice6/1
  Slice5/1 -> [data_buf], Slice8/1
  Slice6/1 -> posedge/1
  [eof_buf] -> eof_buf/1
  posedge/1 -> [eof_buf], Gateway Out9/1
  Gateway Out6/1 -> Scope1/1
  Gateway Out5/1 -> Scope1/2
  Gateway Out7/1 -> Scope1/3
  Gateway Out8/1 -> Scope1/4
  Slice7/1 -> Gateway Out6/1
  [writing_header] -> Gateway Out8/1
  Gateway Out10/1 -> Scope3/1
  Gateway Out9/1 -> Scope3/2
  Gateway Out11/1 -> Scope3/3
  Gateway Out12/1 -> Scope3/4
  Gateway Out14/1 -> Scope3/5
  Slice8/1 -> Gateway Out10/1
  [writing_header] -> Gateway Out14/1
  eof_in/1 -> [eof_in]
  Delay3/1 -> Logical9/2
  Logical9/1 -> [new_chunk]
  heap_size/1 -> Convert/1
  Convert/1 -> Concat6/2
  [new_chunk] -> count_offset/2(en), Register4/2
  Register4/1 -> count_offset/1(sync)

== system x64_32s_001_4ks/pipeline ==
  d : Inport
  Register0 : xbsIndex_r4/Register  init=0  rst=off  en=off  dbl_ovrd=off  block_type=register
      settings: block_version=11.4
  Register1 : xbsIndex_r4/Register  init=0  rst=off  en=off  dbl_ovrd=off  block_type=register
      settings: block_version=11.4
  q : Outport
  wires:
  Register1/1 -> q/1
  Register0/1 -> Register1/1
  d/1 -> Register0/1

== system x64_32s_001_4ks/pipeline1 ==
  d : Inport
  Register0 : xbsIndex_r4/Register  init=0  rst=off  en=off  dbl_ovrd=off  block_type=register
      settings: block_version=11.4
  q : Outport
  wires:
  Register0/1 -> q/1
  d/1 -> Register0/1

== system x64_32s_001_4ks/pipeline10 ==
  d : Inport
  Register0 : xbsIndex_r4/Register  init=0  rst=off  en=off  dbl_ovrd=off  block_type=register
      settings: block_version=11.4
  q : Outport
  wires:
  Register0/1 -> q/1
  d/1 -> Register0/1

== system x64_32s_001_4ks/pipeline11 ==
  d : Inport
  Register0 : xbsIndex_r4/Register  init=0  rst=off  en=off  dbl_ovrd=off  block_type=register
      settings: block_version=11.4
  q : Outport
  wires:
  Register0/1 -> q/1
  d/1 -> Register0/1

== system x64_32s_001_4ks/pipeline12 ==
  d : Inport
  Register0 : xbsIndex_r4/Register  init=0  rst=off  en=off  dbl_ovrd=off  block_type=register
      settings: block_version=11.4
  q : Outport
  wires:
  Register0/1 -> q/1
  d/1 -> Register0/1

== system x64_32s_001_4ks/pipeline13 ==
  d : Inport
  Register0 : xbsIndex_r4/Register  init=0  rst=off  en=off  dbl_ovrd=off  block_type=register
      settings: block_version=11.4
  q : Outport
  wires:
  Register0/1 -> q/1
  d/1 -> Register0/1

== system x64_32s_001_4ks/pipeline14 ==
  d : Inport
  Register0 : xbsIndex_r4/Register  init=0  rst=off  en=off  dbl_ovrd=off  block_type=register
      settings: block_version=11.4
  q : Outport
  wires:
  Register0/1 -> q/1
  d/1 -> Register0/1

== system x64_32s_001_4ks/pipeline15 ==
  d : Inport
  Register0 : xbsIndex_r4/Register  init=0  rst=off  en=off  dbl_ovrd=off  block_type=register
      settings: block_version=11.4
  q : Outport
  wires:
  Register0/1 -> q/1
  d/1 -> Register0/1

== system x64_32s_001_4ks/pipeline16 ==
  d : Inport
  Register0 : xbsIndex_r4/Register  init=0  rst=off  en=off  dbl_ovrd=off  block_type=register
      settings: block_version=11.4
  q : Outport
  wires:
  Register0/1 -> q/1
  d/1 -> Register0/1

== system x64_32s_001_4ks/pipeline17 ==
  d : Inport
  Register0 : xbsIndex_r4/Register  init=0  rst=off  en=off  dbl_ovrd=off  block_type=register
      settings: block_version=11.4
  q : Outport
  wires:
  Register0/1 -> q/1
  d/1 -> Register0/1

== system x64_32s_001_4ks/pipeline18 ==
  d : Inport
  Register0 : xbsIndex_r4/Register  init=0  rst=off  en=off  dbl_ovrd=off  block_type=register
      settings: block_version=11.4
  q : Outport
  wires:
  Register0/1 -> q/1
  d/1 -> Register0/1

== system x64_32s_001_4ks/pipeline19 ==
  d : Inport
  Register0 : xbsIndex_r4/Register  init=0  rst=off  en=off  dbl_ovrd=off  block_type=register
      settings: block_version=11.4
  q : Outport
  wires:
  Register0/1 -> q/1
  d/1 -> Register0/1

== system x64_32s_001_4ks/pipeline2 ==
  d : Inport
  Register0 : xbsIndex_r4/Register  init=0  rst=off  en=off  dbl_ovrd=off  block_type=register
      settings: block_version=11.4
  q : Outport
  wires:
  Register0/1 -> q/1
  d/1 -> Register0/1

== system x64_32s_001_4ks/pipeline20 ==
  d : Inport
  Register0 : xbsIndex_r4/Register  init=0  rst=off  en=off  dbl_ovrd=off  block_type=register
      settings: block_version=11.4
  q : Outport
  wires:
  Register0/1 -> q/1
  d/1 -> Register0/1

== system x64_32s_001_4ks/pipeline21 ==
  d : Inport
  Register0 : xbsIndex_r4/Register  init=0  rst=off  en=off  dbl_ovrd=off  block_type=register
      settings: block_version=11.4
  q : Outport
  wires:
  Register0/1 -> q/1
  d/1 -> Register0/1

== system x64_32s_001_4ks/pipeline22 ==
  d : Inport
  Register0 : xbsIndex_r4/Register  init=0  rst=off  en=off  dbl_ovrd=off  block_type=register
      settings: block_version=11.4
  q : Outport
  wires:
  Register0/1 -> q/1
  d/1 -> Register0/1

== system x64_32s_001_4ks/pipeline23 ==
  d : Inport
  Register0 : xbsIndex_r4/Register  init=0  rst=off  en=off  dbl_ovrd=off  block_type=register
      settings: block_version=11.4
  q : Outport
  wires:
  Register0/1 -> q/1
  d/1 -> Register0/1

== system x64_32s_001_4ks/pipeline24 ==
  d : Inport
  Register0 : xbsIndex_r4/Register  init=0  rst=off  en=off  dbl_ovrd=off  block_type=register
      settings: block_version=11.4
  q : Outport
  wires:
  Register0/1 -> q/1
  d/1 -> Register0/1

== system x64_32s_001_4ks/pipeline3 ==
  d : Inport
  Register0 : xbsIndex_r4/Register  init=0  rst=off  en=off  dbl_ovrd=off  block_type=register
      settings: block_version=11.4
  q : Outport
  wires:
  Register0/1 -> q/1
  d/1 -> Register0/1

== system x64_32s_001_4ks/pipeline4 ==
  d : Inport
  Register0 : xbsIndex_r4/Register  init=0  rst=off  en=off  dbl_ovrd=off  block_type=register
      settings: block_version=11.4
  q : Outport
  wires:
  Register0/1 -> q/1
  d/1 -> Register0/1

== system x64_32s_001_4ks/pipeline5 ==
  d : Inport
  Register0 : xbsIndex_r4/Register  init=0  rst=off  en=off  dbl_ovrd=off  block_type=register
      settings: block_version=11.4
  q : Outport
  wires:
  Register0/1 -> q/1
  d/1 -> Register0/1

== system x64_32s_001_4ks/pipeline6 ==
  d : Inport
  Register0 : xbsIndex_r4/Register  init=0  rst=off  en=off  dbl_ovrd=off  block_type=register
      settings: block_version=11.4
  q : Outport
  wires:
  Register0/1 -> q/1
  d/1 -> Register0/1

== system x64_32s_001_4ks/pipeline7 ==
  d : Inport
  Register0 : xbsIndex_r4/Register  init=0  rst=off  en=off  dbl_ovrd=off  block_type=register
      settings: block_version=11.4
  q : Outport
  wires:
  Register0/1 -> q/1
  d/1 -> Register0/1

== system x64_32s_001_4ks/pipeline8 ==
  d : Inport
  Register0 : xbsIndex_r4/Register  init=0  rst=off  en=off  dbl_ovrd=off  block_type=register
      settings: block_version=11.4
  q : Outport
  wires:
  Register0/1 -> q/1
  d/1 -> Register0/1

== system x64_32s_001_4ks/pipeline9 ==
  d : Inport
  Register0 : xbsIndex_r4/Register  init=0  rst=off  en=off  dbl_ovrd=off  block_type=register
      settings: block_version=11.4
  q : Outport
  wires:
  Register0/1 -> q/1
  d/1 -> Register0/1

== system x64_32s_001_4ks/pow_sim_mux ==
  sync : Inport  OutDataType=fixdt(1, 16)  OutScaling=2^0
  din : Inport  Port=2  OutDataType=fixdt(1, 16)  OutScaling=2^0
  Convert3 : xbsIndex_r4/Convert  arith_type=Unsigned  n_bits=25  bin_pt=24  quantization="Round  (unbiased: Even Values)"  overflow=Wrap  latency=0  dbl_ovrd=off  pipeline=on  block_type=convert
      settings: block_version=11.4
  Counter : xbsIndex_r4/Counter  cnt_type=Free Running  cnt_to=Inf  operation=Up  start_count=0  cnt_by_val=1  arith_type=Unsigned  n_bits=3  bin_pt=0  load_pin=off  rst=on  en=off  explicit_period=on  period=1  dbl_ovrd=off  use_behavioral_HDL=off  implementation=Fabric  block_type=counter
      settings: block_version=11.4
  dout_sim : xbsIndex_r4/Simulation Multiplexer  sim_sel=1  hw_sel=2  block_type=simmux
      settings: infoedit="Distinguish input subsystems as \"simulation only\" and \"generation only\".  The input specified For Simulation will be used during Simulink simulation.  The input specified For Generation will be used during code generation.  This block will typically be used on the output of a HW Co-Simulation, Black Box, or ModelSim subsystem.<br><br>Hardware Notes: This block costs nothing."  block_version=11.4
  dout : Outport
  wires:
  Counter/1 -> Convert3/1
  din/1 -> dout_sim/2
  dout_sim/1 -> dout/1
  sync/1 -> Counter/1
  Convert3/1 -> dout_sim/1
